simulink library "Renault_Library"  (Simulink 9.2)
settings: Solver=ode3  StartTime=0.0  StopTime=10.0  FixedStep=0.020  RelTol=1e-3  EnableMultiTasking=on
undefined symbols (not defined in the file or in its folder): ABC, AssystemProposal, b, chkval, CoeffA_C, CoeffB_C, CoeffMean_C, ConfTime_C, DiagDelay_C, DividePercent, FailureConfActive_C, FallingEdgeDuration_C, HighLevelThresholdVoltage_C, HighLevelVoltage_C, HighLevelVoltageTunable_C, InitValue_C, InputVoltRangeMax_C, InputVoltRangeMin_C, LowActiveContact_C, LowLevelThresholdVoltage_C, LowLevelVoltage_C, MaxOperatingVoltage_C, MinOperatingVoltage_C, mV2V_conversion, OUT1, OutOfRangeHigh_C, OutOfRangeLow_C, PinSupplySaturationMax, PinSupplySaturationMin, Res1Value_C, Res2Value_C, Res3Value_C, RisingEdgeDuration_C, RSA_CANSignalFailMonitoring, SampleTime_C, service14Table, service19Table, service22Table, service24Table, service28Table, service2ETable, service2FTable, service31Table, service87Table, SizeXStateDID2FTable, SizeXTable14, SizeXTable19, SizeXTable22, SizeXTable24, SizeXTable28, SizeXTable2E, SizeXTable2F, SizeXTable31, SizeXTable87, SizeYStateDID2FTable, SizeYTable14, SizeYTable19, SizeYTable22, SizeYTable24, SizeYTable28 ... (+22)
undefined symbols (not defined in the file): BatteryVoltage_ActivationThreshold_C, FrameStatus_AbsentValue_C, FrameStatus_ConfAbsentValue_C, MemThreshold_C, NAMEOFTHEMODEL_SAMPLE_TIME_CST, NbAbs1_C, NbConfAbs1_C, NbConfPres1_C, NbPres1_C, NOMDUSIGNAL_FaultConfTime_C, NOMDUSIGNAL_FaultRehabTime_C, NOMDUSIGNAL_switchvalue_C, NOMDUSIGNAL_unavailablevalue_C, PeriodFrame1_C, ResetTime_C, WakeUpTime_C
libraries: $bdroot x112, simulink x75
(part 10 of 32)

== system Renault_Library/Complex blocks/Math Operations/RSA_DivisionByZero_v01_04 ==
  den : Inport  Tag=AM,In,0,1,Name,C;CMP,Port,PortWidth,SampleTime;
  num : Inport  Tag=AM,In,0,1,Name,C;CMP,Port,PortWidth,SampleTime;  Port=2
  ans_of_0div : Inport  Tag=AM,In,0,1,Name,C;CMP,Port,PortWidth,SampleTime;  Port=3
  Divide : Product  Tag=[LIB]  Inputs=*/  OutDataTypeStr=Inherit: Inherit via back propagation  SaturateOnIntegerOverflow=on  RndMeth=Zero
      settings: AttributesFormatString=SAT = %<SaturateOnIntegerOverflow>
  RelationalOperator : RelationalOperator  Tag=AM,Std,2,1;CMP,Operator;  Operator=~=  OutDataTypeStr=boolean  RndMeth=Nearest  InputSameDT=on
  Switch : Switch  Tag=GEN,Threshold;AM,Switch,2,1;CMP,Threshold;  Criteria=u2 ~= 0  RndMeth=Zero  SaturateOnIntegerOverflow=on  InputSameDT=on
      settings: AttributesFormatString=Criteria=%<Criteria>
  imm_zero : Constant  Tag=AM,Constant,0,1,Value;CMP,Value;  Value=DIVBY0  OutDataTypeStr=Inherit: Inherit via back propagation  SampleTime=-1
  ans : Outport  Tag=AM,Out,1,0,Name,NC;CMP,Port,OutputWhenDisabled,InitialOutput;
  wires:
  den/1 -> RelationalOperator/1, Divide/2
  Switch/1 -> ans/1
  ans_of_0div/1 -> Switch/3
  imm_zero/1 -> RelationalOperator/2
  Divide/1 -> Switch/1
  RelationalOperator/1 -> Switch/2
  num/1 -> Divide/1

== system Renault_Library/Complex blocks/Math Operations/RSA_DivisionByZero_v01_05 ==
  den : Inport
      settings: AttributesFormatString=Type : %<OutDataTypeStr>
  num : Inport  Port=2
      settings: AttributesFormatString=Type : %<OutDataTypeStr>
  ans_of_0div : Inport  Port=3
      settings: AttributesFormatString=Type : %<OutDataTypeStr>
  Abs1 : Abs  OutDataTypeStr=Inherit: Inherit via back propagation  RndMeth=Zero  SaturateOnIntegerOverflow=on
  Divide : Product  Inputs=*/  OutDataTypeStr=Inherit: Inherit via back propagation  SaturateOnIntegerOverflow=on  RndMeth=Zero
      settings: AttributesFormatString=SAT = %<SaturateOnIntegerOverflow>
  RelationalOperator : RelationalOperator  Operator=>  OutDataTypeStr=boolean  RndMeth=Nearest  InputSameDT=on
  Switch : Switch  Criteria=u2 ~= 0  RndMeth=Zero  SaturateOnIntegerOverflow=on  InputSameDT=on
      settings: AttributesFormatString=Criteria=%<Criteria>
  imm_zero : Constant  Value=Den_Tol  OutDataTypeStr=Inherit: Inherit via back propagation
  result : Outport
      settings: AttributesFormatString=Type : %<OutDataTypeStr>\nInit :%<InitialOutput>
  wires:
  Abs1/1 -> RelationalOperator/1
  den/1 -> Abs1/1, Divide/2
  Switch/1 -> result/1
  ans_of_0div/1 -> Switch/3
  imm_zero/1 -> RelationalOperator/2
  Divide/1 -> Switch/1
  RelationalOperator/1 -> Switch/2
  num/1 -> Divide/1

== system Renault_Library/Complex blocks/Math Operations/RSA_Modulo_V01_00 ==
  note: Generate an error if inputs are not of the same data types
  X : Inport
  Y : Inport  Port=2
  Abs1 : Abs  RndMeth=Zero
  Abs2 : Abs  RndMeth=Zero
  Add1 : Sum  Inputs=-+  OutDataTypeStr=Inherit: Inherit via back propagation  RndMeth=Zero  InputSameDT=on
      settings: AttributesFormatString=SAT = %<SaturateOnIntegerOverflow>
  Add2 : Sum  Inputs=+-  OutDataTypeStr=Inherit: Inherit via back propagation  RndMeth=Zero  InputSameDT=on
      settings: AttributesFormatString=SAT = %<SaturateOnIntegerOverflow>
  Constant1 : Constant  Value=cmpTol  OutDataTypeStr=Inherit: Inherit via back propagation
  Constant2 : Constant  Value=cmpZero  OutDataTypeStr=Inherit: Inherit via back propagation
  Constant3 : Constant  Value=constOne  OutDataTypeStr=uint8
  Divide : Product  Inputs=*/  OutDataTypeStr=InternalDataType  SaturateOnIntegerOverflow=on  RndMeth=Zero
  LogicalOperator1 : Logic  OutDataTypeStr=boolean
  LogicalOperator3 : Logic  Operator=XOR  OutDataTypeStr=boolean
  Mux2 : Mux  Inputs=2
  Product1 : Product  OutDataTypeStr=Inherit: Inherit via back propagation  RndMeth=Zero
  Product2 : Product  OutDataTypeStr=Inherit: Inherit via back propagation  SaturateOnIntegerOverflow=on  RndMeth=Zero
  RelationalOperator1 : RelationalOperator  Operator=>  OutDataTypeStr=boolean  RndMeth=Nearest  InputSameDT=on
  RelationalOperator2 : RelationalOperator  Operator=>  OutDataTypeStr=boolean  RndMeth=Nearest  InputSameDT=on
  RelationalOperator5 : RelationalOperator  Operator=<  OutDataTypeStr=boolean  RndMeth=Nearest  InputSameDT=on
  RelationalOperator6 : RelationalOperator  Operator=<  OutDataTypeStr=boolean  RndMeth=Nearest  InputSameDT=on
  Sign : Signum
  Switch : Switch  Criteria=u2 ~= 0  RndMeth=Zero  InputSameDT=on
      settings: AttributesFormatString=Criteria=%<Criteria>
  Switch4 : Switch  Criteria=u2 ~= 0  RndMeth=Zero  InputSameDT=on
      settings: AttributesFormatString=Criteria=%<Criteria>
  Terminator1 : Terminator
  Terminator2 : Terminator
  Terminator3 : Terminator
  M : Outport
  wires:
  Add2/1 -> Switch4/1
  RelationalOperator1/1 -> Switch/2
  [absY] -> Add2/1
  [absX] -> Add1/2
  [absY] -> Product2/2
  Abs2/1 -> [absY]
  Abs1/1 -> [absX]
  Y/1 -> Abs2/1, [Y]
  X/1 -> [X], Abs1/1
  [cmpzero] -> RelationalOperator5/2
  [cmpzero] -> RelationalOperator6/2
  [Y] -> RelationalOperator5/1
  [X] -> RelationalOperator6/1
  RelationalOperator6/1 -> LogicalOperator3/1
  RelationalOperator5/1 -> LogicalOperator3/2
  Product2/1 -> Add1/1
  [X] -> Switch/3
  Switch/1 -> M/1
  [absX] -> Divide/1
  [absY] -> Divide/2
  [absY] -> RelationalOperator1/1
  [Y] -> Mux2/2
  [X] -> Mux2/1
  Mux2/1 -> Terminator3/1
  Constant1/1 -> [cmpTol]
  [cmpTol] -> RelationalOperator1/2
  Divide/1 -> Product2/1
  Add1/1 -> Add2/2, RelationalOperator2/1, Switch4/3
  Constant2/1 -> [cmpzero]
  [cmpzero] -> RelationalOperator2/2
  RelationalOperator2/1 -> LogicalOperator1/2
  LogicalOperator3/1 -> LogicalOperator1/1
  LogicalOperator1/1 -> Switch4/2
  [Y] -> Sign/1
  Sign/1 -> [Mulconst]
  Constant3/1 -> [constone]
  [constone] -> Terminator1/1
  [Mulconst] -> Terminator2/1
  [Mulconst] -> Product1/2
  Switch4/1 -> Product1/1
  Product1/1 -> Switch/1

== system Renault_Library/Complex blocks/Signal Routing/RSA_DataTypeDuplicate ==
  In1 : Inport
      settings: AttributesFormatString=Type : %<OutDataType>\nScaling : %<OutScaling>
  In2 : Inport  Port=2
      settings: AttributesFormatString=Type : %<OutDataType>\nScaling : %<OutScaling>
  In3 : Inport  Port=3
      settings: AttributesFormatString=Type : %<OutDataType>\nScaling : %<OutScaling>
  Mux : Mux  Inputs=3
  Terminator : Terminator
  wires:
  In3/1 -> Mux/3
  In2/1 -> Mux/2
  In1/1 -> Mux/1
  Mux/1 -> Terminator/1

== system Renault_Library/Complex blocks/Signal Routing/RSA_DataTypeDuplicate_v01_03 ==
  In1 : Inport
  In2 : Inport  Port=2
  In3 : Inport  Port=3
  Mux : Mux  Inputs=3
  Terminator : Terminator
  wires:
  Mux/1 -> Terminator/1
  In1/1 -> Mux/1
  In2/1 -> Mux/2
  In3/1 -> Mux/3

== system Renault_Library/Complex blocks/Signal Routing/RSA_SlopeSwitch2Inputs ==
  Switch_Cmd : Inport  Tag=AM,In,0,1,Name,C;CMP,Port,PortWidth,SampleTime;
      settings: AttributesFormatString=Type : %<OutDataTypeStr>
  Pos_Slope : Inport  Port=2
      settings: AttributesFormatString=Type : %<OutDataTypeStr>
  Neg_Slope : Inport  Port=3
      settings: AttributesFormatString=Type : %<OutDataTypeStr>
  In0 : Inport  Port=4
      settings: AttributesFormatString=Type : %<OutDataTypeStr>
  In1 : Inport  Port=5
      settings: AttributesFormatString=Type : %<OutDataTypeStr>
  Constant : Constant  Value=STHCMD  OutDataTypeStr=Inherit: Inherit via back propagation  SampleTime=-1
  Constant1 : Constant  Value=st  OutDataTypeStr=Inherit: Inherit via back propagation  SampleTime=-1
  DataTypeConversion : DataTypeConversion  RndMeth=Zero
  DataTypeConversion1 : DataTypeConversion  RndMeth=Zero
  Multiport_Switch : MultiPortSwitch  Inputs=2  RndMeth=Zero  SaturateOnIntegerOverflow=on  InputSameDT=on
      settings: AttributesFormatString=PortOrder=%<DataPortOrder>
  Product : Product  OutDataTypeStr=Inherit: Inherit via back propagation  SaturateOnIntegerOverflow=on  RndMeth=Zero
      settings: AttributesFormatString=SAT = %<SaturateOnIntegerOverflow>
  Product1 : Product  OutDataTypeStr=Inherit: Inherit via back propagation  SaturateOnIntegerOverflow=on  RndMeth=Zero
      settings: AttributesFormatString=SAT = %<SaturateOnIntegerOverflow>
  Signal_Change_Compute : Subsystem
  Slope_On_Block : Subsystem
  Slope_On_Compute : Subsystem
  Sum : Sum  OutDataTypeStr=Inherit: Inherit via back propagation  RndMeth=Zero  InputSameDT=on
      settings: AttributesFormatString=SAT = %<SaturateOnIntegerOverflow>
  Switch2 : Switch  Tag=GEN,Threshold;AM,Switch,2,1;CMP,Threshold;  Criteria=u2 ~= 0  RndMeth=Zero  SaturateOnIntegerOverflow=on  InputSameDT=on
      settings: AttributesFormatString=Criteria=%<Criteria>
  UnitDelay1 : UnitDelay  InitialCondition=false  SampleTime=-1
  UnitDelay2 : UnitDelay  InitialCondition=SS2ININI  SampleTime=-1
  Output : Outport  Tag=AM,Out,1,0,Name,NC;CMP,Port,OutputWhenDisabled,InitialOutput;
      settings: AttributesFormatString=Type : %<OutDataTypeStr>\nInit :%<InitialOutput>
  Slope_On : Outport  Tag=AM,Out,1,0,Name,NC;CMP,Port,OutputWhenDisabled,InitialOutput;  Port=2
      settings: AttributesFormatString=Type : %<OutDataTypeStr>\nInit :%<InitialOutput>
  wires:
  DataTypeConversion1/1 -> Output/1
  DataTypeConversion/1 -> Slope_On/1
  Switch_Cmd/1 -> Sum/1
  Sum/1 -> Signal_Change_Compute/1(Switch_Cmd), Multiport_Switch/1
  Constant/1 -> Sum/2
  Pos_Slope/1 -> [Pos_Slope]
  Neg_Slope/1 -> [Neg_Slope]
  [Enable_Slope_On_Block] -> Switch2/2
  [Output_Not_Equals_Input] -> UnitDelay1/1
  Slope_On_Block/1(Output_Not_Equals_Input) -> [Output_Not_Equals_Input]
  Constant1/1 -> Product1/2, Product/2
  Multiport_Switch/1 -> [Input]
  [Input] -> Switch2/3
  [Input] -> Slope_On_Block/2(Input)
  Signal_Change_Compute/1(Signal_Change) -> Slope_On_Compute/1(Signal_Change), Slope_On_Block/1(Signal_Change)
  [Neg_Slope] -> Product1/1
  [Pos_Slope] -> Product/1
  Product1/1 -> Slope_On_Block/4(Neg_Slope)
  Product/1 -> Slope_On_Block/3(Pos_Slope)
  UnitDelay2/1 -> Slope_On_Block/5(Prev_Output)  "X2"
  UnitDelay1/1 -> Slope_On_Compute/2(Output_Not_Equals_Input)
  Switch2/1 -> DataTypeConversion1/1, UnitDelay2/1
  Slope_On_Block/2(Output) -> Switch2/1  "X1"
  Slope_On_Compute/1(Slope_On) -> [Enable_Slope_On_Block], Slope_On_Block/Enable, DataTypeConversion/1
  In0/1 -> Multiport_Switch/2
  In1/1 -> Multiport_Switch/3

== system Renault_Library/Complex blocks/Timers & Counters/RSA_IncAndDecCounter ==
  INC_COUNTER : Inport
      settings: AttributesFormatString=Type : %<OutDataTypeStr>
  DEC_COUNTER : Inport  Port=2
      settings: AttributesFormatString=Type : %<OutDataTypeStr>
  MAX_COUNTER : Inport  Port=3
      settings: AttributesFormatString=Type : %<OutDataTypeStr>
  MIN_COUNTER : Inport  Port=4
      settings: AttributesFormatString=Type : %<OutDataTypeStr>
  RESET_COUNTER : Inport  Port=5
      settings: AttributesFormatString=Type : %<OutDataTypeStr>
  COUNTER_STEP : Inport  Port=6
      settings: AttributesFormatString=Type : %<OutDataTypeStr>
  INIT_COUNTER : Inport  Port=7
      settings: AttributesFormatString=Type : %<OutDataTypeStr>
  Add1 : Sum  OutDataTypeStr=Inherit: Inherit via back propagation  RndMeth=Zero  SaturateOnIntegerOverflow=on  InputSameDT=on
      settings: AttributesFormatString=SAT = %<SaturateOnIntegerOverflow>
  Add2 : Sum  Inputs=+-  OutDataTypeStr=Inherit: Inherit via back propagation  RndMeth=Zero  SaturateOnIntegerOverflow=on  InputSameDT=on
      settings: AttributesFormatString=SAT = %<SaturateOnIntegerOverflow>
  Constant1 : Constant  Value=4  OutDataTypeStr=Inherit: Inherit via back propagation  SampleTime=-1
  Constant2 : Constant  Value=2  OutDataTypeStr=Inherit: Inherit via back propagation  SampleTime=-1
  Constant3 : Constant  Value=0  OutDataTypeStr=Inherit: Inherit via back propagation  SampleTime=-1
  Constant5 : Constant  Value=3  OutDataTypeStr=Inherit: Inherit via back propagation  SampleTime=-1
  Constant6 : Constant  OutDataTypeStr=uint8  SampleTime=-1
  Data_Type_Conversion : DataTypeConversion  RndMeth=Zero
  MinMax : MinMax  Inputs=2  OutDataTypeStr=Inherit: Inherit via back propagation  RndMeth=Zero  InputSameDT=on
  MinMax1 : MinMax  Function=max  Inputs=2  OutDataTypeStr=Inherit: Inherit via back propagation  RndMeth=Zero  InputSameDT=on
  Multiport_Switch : MultiPortSwitch  Inputs=4  RndMeth=Zero  InputSameDT=on
      settings: AttributesFormatString=PortOrder=%<DataPortOrder>
  Switch{,2..4} (4 alike) : Switch  Criteria=u2 ~= 0  RndMeth=Zero  InputSameDT=on
      settings: AttributesFormatString=Criteria=%<Criteria>
  UnitDelay : UnitDelay  InitialCondition=true  SampleTime=-1
      settings: AttributesFormatString=IC = %<X0>
  Unit_Delay1 : UnitDelay  SampleTime=-1
      settings: AttributesFormatString=IC = %<X0>
  true : Constant  Value=false  OutDataTypeStr=boolean  SampleTime=-1
  COUNTER_OUT : Outport
      settings: AttributesFormatString=Type : %<OutDataTypeStr>\nInit :%<InitialOutput>
  wires:
  [MIN_COUNTER] -> MinMax1/2
  MinMax/1 -> MinMax1/1
  Multiport_Switch/1 -> MinMax/1
  [MAX_COUNTER] -> MinMax/2
  Constant2/1 -> Switch2/1
  Constant6/1 -> Switch4/1
  Switch2/1 -> Switch4/3
  Switch4/1 -> [SELECT_COUNTER]
  [SELECT_COUNTER] -> Multiport_Switch/1
  Add1/1 -> Multiport_Switch/2
  Add2/1 -> Multiport_Switch/3
  [COUNTER_STEP] -> Add2/2
  [COUNTER_STEP] -> Add1/2
  Unit_Delay1/1 -> Add1/1, Add2/1, Multiport_Switch/5
  MAX_COUNTER/1 -> [MAX_COUNTER]
  MIN_COUNTER/1 -> [MIN_COUNTER]
  INC_COUNTER/1 -> Switch4/2
  DEC_COUNTER/1 -> Switch2/2
  Constant5/1 -> Switch3/1
  Constant1/1 -> Switch3/3
  Switch3/1 -> Switch2/3
  RESET_COUNTER/1 -> Switch3/2
  Constant3/1 -> Multiport_Switch/4
  COUNTER_STEP/1 -> [COUNTER_STEP]
  Data_Type_Conversion/1 -> COUNTER_OUT/1
  true/1 -> UnitDelay/1
  INIT_COUNTER/1 -> [INIT_COUNTER]
  [INIT_COUNTER] -> Switch/1
  MinMax1/1 -> Switch/3
  Switch/1 -> Unit_Delay1/1, Data_Type_Conversion/1
  UnitDelay/1 -> Switch/2

== system Renault_Library/Complex blocks/Timers & Counters/RSA_IncAndDecCounter_V01_01 ==
  INC_COUNTER : Inport
      settings: AttributesFormatString=Type : %<OutDataTypeStr>
  DEC_COUNTER : Inport  Port=2
      settings: AttributesFormatString=Type : %<OutDataTypeStr>
  MAX_COUNTER : Inport  Port=3
      settings: AttributesFormatString=Type : %<OutDataTypeStr>
  MIN_COUNTER : Inport  Port=4
      settings: AttributesFormatString=Type : %<OutDataTypeStr>
  RESET_COUNTER : Inport  Port=5
      settings: AttributesFormatString=Type : %<OutDataTypeStr>
  COUNTER_STEP : Inport  Port=6
      settings: AttributesFormatString=Type : %<OutDataTypeStr>
  INIT_COUNTER : Inport  Port=7
      settings: AttributesFormatString=Type : %<OutDataTypeStr>
  Add1 : Sum  OutDataTypeStr=Inherit: Inherit via back propagation  RndMeth=Zero  SaturateOnIntegerOverflow=on  InputSameDT=on
      settings: AttributesFormatString=SAT = %<SaturateOnIntegerOverflow>
  Add2 : Sum  Inputs=+-  OutDataTypeStr=Inherit: Inherit via back propagation  RndMeth=Zero  SaturateOnIntegerOverflow=on  InputSameDT=on
      settings: AttributesFormatString=SAT = %<SaturateOnIntegerOverflow>
  Constant2 : Constant  Value=CONS_TWO  OutDataTypeStr=Inherit: Inherit via back propagation
  Constant3 : Constant  Value=CONS_ZERO  OutDataTypeStr=Inherit: Inherit via back propagation
  Constant4 : Constant  Value=CONS_ZERO  OutDataTypeStr=uint8
  Constant5 : Constant  Value=CONS_THREE  OutDataTypeStr=Inherit: Inherit via back propagation
  Constant6 : Constant  Value=CONS_ONE  OutDataTypeStr=uint8
  Data_Type_Conversion : DataTypeConversion  RndMeth=Zero
  MinMax : MinMax  Inputs=2  OutDataTypeStr=Inherit: Inherit via back propagation  RndMeth=Zero  InputSameDT=on
  MinMax1 : MinMax  Function=max  Inputs=2  OutDataTypeStr=Inherit: Inherit via back propagation  RndMeth=Zero  InputSameDT=on
  Multiport_Switch : MultiPortSwitch  DataPortOrder=Zero-based contiguous  Inputs=4  zeroidx=on  RndMeth=Zero  InputSameDT=on
      settings: AttributesFormatString=PortOrder=%<DataPortOrder>
  Switch{,2..4} (4 alike) : Switch  Criteria=u2 ~= 0  RndMeth=Zero  InputSameDT=on
      settings: AttributesFormatString=Criteria=%<Criteria>
  UnitDelay : UnitDelay  InitialCondition=true  SampleTime=-1
      settings: AttributesFormatString=IC = %<X0>
  Unit_Delay1 : UnitDelay  SampleTime=-1
      settings: AttributesFormatString=IC = %<X0>
  true : Constant  Value=false  OutDataTypeStr=boolean
  COUNTER_OUT : Outport
      settings: AttributesFormatString=Type : %<OutDataTypeStr>\nInit :%<InitialOutput>
  wires:
  Constant5/1 -> Switch3/3
  Constant2/1 -> Switch3/1
  Constant6/1 -> Switch2/1
  Constant4/1 -> Switch4/1
  [MIN_COUNTER] -> MinMax1/2
  MinMax/1 -> MinMax1/1
  Multiport_Switch/1 -> MinMax/1
  [MAX_COUNTER] -> MinMax/2
  Switch2/1 -> Switch4/3
  Switch4/1 -> [SELECT_COUNTER]
  [SELECT_COUNTER] -> Multiport_Switch/1
  Add1/1 -> Multiport_Switch/2
  Add2/1 -> Multiport_Switch/3
  [COUNTER_STEP] -> Add2/2
  [COUNTER_STEP] -> Add1/2
  Unit_Delay1/1 -> Add1/1, Add2/1, Multiport_Switch/5
  MAX_COUNTER/1 -> [MAX_COUNTER]
  MIN_COUNTER/1 -> [MIN_COUNTER]
  INC_COUNTER/1 -> Switch4/2
  DEC_COUNTER/1 -> Switch2/2
  Switch3/1 -> Switch2/3
  RESET_COUNTER/1 -> Switch3/2
  Constant3/1 -> Multiport_Switch/4
  COUNTER_STEP/1 -> [COUNTER_STEP]
  Data_Type_Conversion/1 -> COUNTER_OUT/1
  true/1 -> UnitDelay/1
  INIT_COUNTER/1 -> [INIT_COUNTER]
  [INIT_COUNTER] -> Switch/1
  MinMax1/1 -> Switch/3
  Switch/1 -> Unit_Delay1/1, Data_Type_Conversion/1
  UnitDelay/1 -> Switch/2

== system Renault_Library/Complex blocks/Timers & Counters/RSA_Timer ==
  Init : Inport
      settings: AttributesFormatString=Type : %<OutDataTypeStr>
  Duration : Inport  Port=2
      settings: AttributesFormatString=Type : %<OutDataTypeStr>
  Constant1 : Constant  Tag=AM,Constant,0,1,Value;CMP,Value;  Value=TIM1INI  OutDataTypeStr=Inherit: Inherit via back propagation  SampleTime=-1
  Constant2 : Constant  Tag=AM,Constant,0,1,Value;CMP,Value;  Value=TIM1INI  OutDataTypeStr=Inherit: Inherit via back propagation  SampleTime=-1
  Constant3 : Constant  Tag=AM,Constant,0,1,Value;CMP,Value;  Value=st  OutDataTypeStr=Inherit: Inherit via back propagation  SampleTime=-1
  Constant4 : Constant  Tag=AM,Constant,0,1,Value;CMP,Value;  Value=TIM1INI  OutDataTypeStr=Inherit: Inherit via back propagation  SampleTime=-1
  DataTypeConversion : DataTypeConversion  RndMeth=Zero
  DelayInput1 : UnitDelay  Description=\nStore in Global RAM  InitialCondition=timeoutinit  SampleTime=-1
  DelayInput2 : UnitDelay  Description=\nStore in Global RAM  InitialCondition=TIM1INI  SampleTime=-1
  EdgeRising : Subsystem  mask(EdgeRising){vinit=initinit}
      mask prompts: vinit "Initialisation"
      mask help: When a rising edge is used as input, the ouput is equal to one during the fall of the signal. Otherwise the output is zero.
      mask Display:
      | plot([0.2 0.4 0.4 0.6],[0.6 0.6 0.9 0.9]);
      | plot([0.2 0.4 0.4 0.4 0.6],[0.1 0.1 0.4 0.1 0.1])
  LogicalOperator : Logic  OutDataTypeStr=boolean
  RelationalOperator1 : RelationalOperator  OutDataTypeStr=boolean  ZeroCross=off  RndMeth=Nearest  InputSameDT=on
  RelationalOperator2 : RelationalOperator  Operator=<=  OutDataTypeStr=boolean  ZeroCross=off  RndMeth=Nearest  InputSameDT=on
  Sum : Sum  Inputs=+-  OutDataTypeStr=Inherit: Inherit via back propagation  RndMeth=Zero  SaturateOnIntegerOverflow=on  InputSameDT=on
      settings: AttributesFormatString=SAT = %<SaturateOnIntegerOverflow>
  Switch{1..4} (4 alike) : Switch  Tag=GEN,Threshold;AM,Switch,2,1;CMP,Threshold;  Criteria=u2 ~= 0  RndMeth=Zero  SaturateOnIntegerOverflow=on  InputSameDT=on
      settings: AttributesFormatString=Criteria=%<Criteria>
  Timeout : Outport
      settings: AttributesFormatString=Type : %<OutDataTypeStr>\nInit :%<InitialOutput>
  Timer : Outport  Port=2
      settings: AttributesFormatString=Type : %<OutDataTypeStr>\nInit :%<InitialOutput>
  wires:
  Constant2/1 -> Switch4/3
  DelayInput2/1 -> RelationalOperator2/2, Sum/1  "X2"
  RelationalOperator2/1 -> Switch4/2
  Sum/1 -> Switch4/1
  Switch4/1 -> Switch2/3
  Duration/1 -> Switch2/1
  [EdgeRising] -> Switch2/2
  Constant3/1 -> Sum/2, RelationalOperator2/1
  Switch3/1 -> DelayInput2/1, DataTypeConversion/1  "X5"
  Constant1/1 -> Switch3/1
  RelationalOperator1/1 -> LogicalOperator/2, Switch3/2  "X4"
  DelayInput1/1 -> Switch1/3
  Switch1/1 -> LogicalOperator/1, DelayInput1/1  "Flag_of_first_initialisation"
  LogicalOperator/1 -> Timeout/1
  Constant4/1 -> RelationalOperator1/1
  Switch2/1 -> Switch3/3, RelationalOperator1/2  "X3"
  Init/1 -> EdgeRising/1(In)
  EdgeRising/1(Out) -> [EdgeRising], Switch1/1, Switch1/2
  DataTypeConversion/1 -> Timer/1

== system Renault_Library/Complex blocks/Timers & Counters/RSA_Timer1 ==
  Init : Inport
      settings: AttributesFormatString=Type : %<OutDataTypeStr>
  Duration : Inport  Port=2
      settings: AttributesFormatString=Type : %<OutDataTypeStr>
  Timestep : Inport  Port=3
      settings: AttributesFormatString=Type : %<OutDataTypeStr>
  Constant1 : Constant  Tag=AM,Constant,0,1,Value;CMP,Value;  Value=TIM1INI  OutDataTypeStr=Inherit: Inherit via back propagation  SampleTime=-1
  Constant2 : Constant  Tag=AM,Constant,0,1,Value;CMP,Value;  Value=TIM1INI  OutDataTypeStr=Inherit: Inherit via back propagation  SampleTime=-1
  Constant4 : Constant  Tag=AM,Constant,0,1,Value;CMP,Value;  Value=TIM1INI  OutDataTypeStr=Inherit: Inherit via back propagation  SampleTime=-1
  DataTypeConversion : DataTypeConversion  RndMeth=Zero
  DelayInput1 : UnitDelay  Description=\nStore in Global RAM  InitialCondition=timeoutinit  SampleTime=-1
  DelayInput2 : UnitDelay  Description=\nStore in Global RAM  InitialCondition=TIM1INI  SampleTime=-1
  EdgeRising : Subsystem  mask(EdgeRising){vinit=initinit}
      mask prompts: vinit "Initialisation"
      mask help: When a rising edge is used as input, the ouput is equal to one during the fall of the signal. Otherwise the output is zero.
      mask Display:
      | plot([0.2 0.4 0.4 0.6],[0.6 0.6 0.9 0.9]);
      | plot([0.2 0.4 0.4 0.4 0.6],[0.1 0.1 0.4 0.1 0.1])
  LogicalOperator : Logic  OutDataTypeStr=boolean
  RelationalOperator1 : RelationalOperator  OutDataTypeStr=boolean  ZeroCross=off  RndMeth=Nearest  InputSameDT=on
  RelationalOperator2 : RelationalOperator  Operator=<=  OutDataTypeStr=boolean  ZeroCross=off  RndMeth=Nearest  InputSameDT=on
  Sum : Sum  Inputs=+-  OutDataTypeStr=Inherit: Inherit via back propagation  RndMeth=Zero  SaturateOnIntegerOverflow=on  InputSameDT=on
      settings: AttributesFormatString=SAT = %<SaturateOnIntegerOverflow>
  Switch{1..4} (4 alike) : Switch  Tag=GEN,Threshold;AM,Switch,2,1;CMP,Threshold;  Criteria=u2 ~= 0  RndMeth=Zero  SaturateOnIntegerOverflow=on  InputSameDT=on
      settings: AttributesFormatString=Criteria=%<Criteria>
  Timeout : Outport
      settings: AttributesFormatString=Type : %<OutDataTypeStr>\nInit :%<InitialOutput>
  Timer : Outport  Port=2
      settings: AttributesFormatString=Type : %<OutDataTypeStr>\nInit :%<InitialOutput>
  wires:
  [Timestep] -> RelationalOperator2/1, Sum/2
  [EdgeRising] -> Switch2/2
  EdgeRising/1(Out) -> Switch1/2, Switch1/1, [EdgeRising]
  Init/1 -> EdgeRising/1(In)
  Constant4/1 -> RelationalOperator1/1
  LogicalOperator/1 -> Timeout/1
  Switch1/1 -> DelayInput1/1, LogicalOperator/1
  DelayInput1/1 -> Switch1/3
  Timestep/1 -> [Timestep]
  RelationalOperator1/1 -> Switch3/2, LogicalOperator/2
  Constant1/1 -> Switch3/1
  Switch3/1 -> DelayInput2/1, DataTypeConversion/1
  DataTypeConversion/1 -> Timer/1
  Switch2/1 -> RelationalOperator1/2, Switch3/3
  Duration/1 -> Switch2/1
  Switch4/1 -> Switch2/3
  Sum/1 -> Switch4/1
  RelationalOperator2/1 -> Switch4/2
  DelayInput2/1 -> Sum/1, RelationalOperator2/2
  Constant2/1 -> Switch4/3

== system Renault_Library/Complex blocks/Timers & Counters/RSA_Timer1_v01_04 ==
  Init : Inport
      settings: AttributesFormatString=Type : %<OutDataTypeStr>
  Duration : Inport  Port=2
      settings: AttributesFormatString=Type : %<OutDataTypeStr>
  Timestep : Inport  Port=3
      settings: AttributesFormatString=Type : %<OutDataTypeStr>
  Constant1 : Constant  Tag=AM,Constant,0,1,Value;CMP,Value;  Value=TIM1INI  OutDataTypeStr=Inherit: Inherit via back propagation  SampleTime=-1
  Constant3 : Constant  Tag=AM,Constant,0,1,Value;CMP,Value;  Value=TIM1INI  OutDataTypeStr=Inherit: Inherit via back propagation  SampleTime=-1
  DataTypeConversion : DataTypeConversion  RndMeth=Zero
  DelayInput1 : UnitDelay  Description=\nStore in Global RAM  InitialCondition=timeoutinit  SampleTime=-1
  DelayInput2 : UnitDelay  Description=\nStore in Global RAM  InitialCondition=TIM1INI  SampleTime=-1
  EdgeRising : Subsystem  mask(EdgeRising){vinit=initinit}
      mask prompts: vinit "Initialisation"
      mask help: When a rising edge is used as input, the ouput is equal to one during the fall of the signal. Otherwise the output is zero.
      mask Display:
      | plot([0.2 0.4 0.4 0.6],[0.6 0.6 0.9 0.9]);
      | plot([0.2 0.4 0.4 0.4 0.6],[0.1 0.1 0.4 0.1 0.1])
  LogicalOperator : Logic  OutDataTypeStr=boolean
  RelationalOperator1 : RelationalOperator  Operator=>  OutDataTypeStr=boolean  ZeroCross=off  RndMeth=Nearest  InputSameDT=on
  RelationalOperator2 : RelationalOperator  Operator=<=  OutDataTypeStr=boolean  ZeroCross=off  RndMeth=Nearest  InputSameDT=on
  Sum : Sum  Inputs=+-  OutDataTypeStr=Inherit: Inherit via back propagation  RndMeth=Zero  SaturateOnIntegerOverflow=on  InputSameDT=on
      settings: AttributesFormatString=SAT = %<SaturateOnIntegerOverflow>
  Switch{1..4} (4 alike) : Switch  Tag=GEN,Threshold;AM,Switch,2,1;CMP,Threshold;  Criteria=u2 ~= 0  RndMeth=Zero  SaturateOnIntegerOverflow=on  InputSameDT=on
      settings: AttributesFormatString=Criteria=%<Criteria>
  Timeout : Outport
      settings: AttributesFormatString=Type : %<OutDataTypeStr>\nInit :%<InitialOutput>
  Timer : Outport  Port=2
      settings: AttributesFormatString=Type : %<OutDataTypeStr>\nInit :%<InitialOutput>
  wires:
  [Timestep] -> RelationalOperator2/1, Sum/2
  [EdgeRising] -> Switch2/2
  EdgeRising/1(Out) -> Switch1/2, Switch1/1, [EdgeRising]
  Init/1 -> EdgeRising/1(In)
  LogicalOperator/1 -> Timeout/1
  Switch1/1 -> DelayInput1/1, LogicalOperator/1
  DelayInput1/1 -> Switch1/3
  Timestep/1 -> [Timestep]
  RelationalOperator1/1 -> LogicalOperator/2, Switch3/2
  Switch3/1 -> DataTypeConversion/1, DelayInput2/1
  DataTypeConversion/1 -> Timer/1
  Switch2/1 -> Switch3/3, RelationalOperator1/2
  Switch4/1 -> Switch2/3
  Sum/1 -> Switch4/1
  RelationalOperator2/1 -> Switch4/2
  DelayInput2/1 -> Sum/1, RelationalOperator2/2
  Duration/1 -> Switch2/1
  [Timestep] -> RelationalOperator1/1
  Constant3/1 -> Switch3/1
  Constant1/1 -> Switch4/3

== system Renault_Library/Complex blocks/Timers & Counters/RSA_Timer1_v01_05 ==
  Init : Inport
      settings: AttributesFormatString=Type : %<OutDataTypeStr>
  Duration : Inport  Port=2
      settings: AttributesFormatString=Type : %<OutDataTypeStr>
  Timestep : Inport  Port=3
      settings: AttributesFormatString=Type : %<OutDataTypeStr>
  Constant1 : Constant  Tag=AM,Constant,0,1,Value;CMP,Value;  Value=TIM1INI  OutDataTypeStr=Inherit: Inherit via back propagation  SampleTime=-1
  Constant3 : Constant  Tag=AM,Constant,0,1,Value;CMP,Value;  Value=TIM1INI  OutDataTypeStr=Inherit: Inherit via back propagation  SampleTime=-1
  Constant4 : Constant  Tag=AM,Constant,0,1,Value;CMP,Value;  Value=1000  OutDataTypeStr=uint16  SampleTime=-1
  Constant5 : Constant  Tag=AM,Constant,0,1,Value;CMP,Value;  OutDataTypeStr=Inherit: Inherit via back propagation  SampleTime=-1
  DataTypeConversion : DataTypeConversion  RndMeth=Zero
  DataTypeConversion1 : DataTypeConversion  OutDataTypeStr=uint32  SaturateOnIntegerOverflow=on  RndMeth=Zero
  DataTypeConversion2 : DataTypeConversion  RndMeth=Zero
  DelayInput1 : UnitDelay  Description=\nStore in Global RAM  InitialCondition=timeoutinit  SampleTime=-1
      settings: AttributesFormatString=IC = %<X0>
  DelayInput2 : UnitDelay  Description=\nStore in Global RAM  InitialCondition=TIM1INI  SampleTime=-1
      settings: AttributesFormatString=IC = %<X0>
  EdgeRising : Subsystem  mask(EdgeRising){vinit=initinit}
      mask prompts: vinit "Initialisation"
      mask help: When a rising edge is used as input, the ouput is equal to one during the fall of the signal. Otherwise the output is zero.
      mask Display:
      | plot([0.2 0.4 0.4 0.6],[0.6 0.6 0.9 0.9]);
      | plot([0.2 0.4 0.4 0.4 0.6],[0.1 0.1 0.4 0.1 0.1])
  LogicalOperator : Logic  OutDataTypeStr=boolean
  Product : Product  SaturateOnIntegerOverflow=on  RndMeth=Zero
  Product1 : Product  SaturateOnIntegerOverflow=on  RndMeth=Zero
  Product2 : Product  Inputs=*/  OutDataTypeStr=Inherit: Inherit via back propagation  SaturateOnIntegerOverflow=on  RndMeth=Zero
  RSA_CompareToZero : $bdroot/Complex blocks/Logic & Bit Operations/RSA_CompareToZero  Tag=[LIB]  SystemSampleTime=-1  relop=~=  LogicOutDataTypeMode=boolean
      settings: AttributesFormatString=%<MaskType> : Ver : 01.03\nOperator: %<relop>\nOutput data type mode: %<LogicOutDataTypeMode>\nEnable zero-crossing detection %<ZeroCross>
  RSA_DataTypeDuplicate_v01_03 : $bdroot/Complex blocks/Signal Routing/RSA_DataTypeDuplicate_v01_03  Tag=[LIB]  SystemSampleTime=-1  NoIn=2
      settings: AttributesFormatString=%<MaskType> : Ver : 01.03\nNo Of Inputs %<NoIn>
  RelationalOperator1 : RelationalOperator  Operator=>  OutDataTypeStr=boolean  ZeroCross=off  RndMeth=Nearest  InputSameDT=on
  RelationalOperator2 : RelationalOperator  Operator=<=  OutDataTypeStr=boolean  ZeroCross=off  RndMeth=Nearest  InputSameDT=on
  Subtract4 : Sum  Inputs=-+  OutDataTypeStr=Inherit: Inherit via back propagation  RndMeth=Zero  SaturateOnIntegerOverflow=on  InputSameDT=on
      settings: AttributesFormatString=SAT = %<SaturateOnIntegerOverflow>
  Sum : Sum  Inputs=+-  OutDataTypeStr=Inherit: Inherit via back propagation  RndMeth=Zero  SaturateOnIntegerOverflow=on  InputSameDT=on
      settings: AttributesFormatString=SAT = %<SaturateOnIntegerOverflow>
  Switch{1..5} (5 alike) : Switch  Tag=GEN,Threshold;AM,Switch,2,1;CMP,Threshold;  Criteria=u2 ~= 0  RndMeth=Zero  SaturateOnIntegerOverflow=on  InputSameDT=on
      settings: AttributesFormatString=Criteria=%<Criteria>
  Timeout : Outport
      settings: AttributesFormatString=Type : %<OutDataTypeStr>\nInit :%<InitialOutput>
  Timer : Outport  Port=2
      settings: AttributesFormatString=Type : %<OutDataTypeStr>\nInit :%<InitialOutput>
  wires:
  [Timestep1] -> RelationalOperator2/1, Sum/2
  [EdgeRising] -> Switch2/2
  EdgeRising/1(Out) -> Switch1/2, Switch1/1, [EdgeRising]
  Init/1 -> EdgeRising/1(In)
  LogicalOperator/1 -> Timeout/1
  Switch1/1 -> DelayInput1/1, LogicalOperator/1
  DelayInput1/1 -> Switch1/3
  RelationalOperator1/1 -> LogicalOperator/2, Switch3/2
  Switch3/1 -> DelayInput2/1, Product2/1
  DataTypeConversion/1 -> Timer/1
  Switch2/1 -> Switch3/3, RelationalOperator1/2
  Switch4/1 -> Switch2/3
  Sum/1 -> Switch4/1
  RelationalOperator2/1 -> Switch4/2
  DelayInput2/1 -> Sum/1, RelationalOperator2/2
  [Timestep1] -> RelationalOperator1/1
  Constant3/1 -> Switch3/1
  Constant1/1 -> Switch4/3
  [Timestep] -> DataTypeConversion1/1, Subtract4/2
  DataTypeConversion1/1 -> DataTypeConversion2/1
  DataTypeConversion2/1 -> Subtract4/1
  RSA_CompareToZero/1 -> Switch5/2
  Subtract4/1 -> RSA_CompareToZero/1
  Constant5/1 -> Switch5/3
  Constant4/1 -> Switch5/1
  Switch5/1 -> [tolcons]
  [tolcons] -> Product/2
  Product/1 -> [Timestep1]
  Duration/1 -> RSA_DataTypeDuplicate_v01_03/2, Product1/1
  [tolcons] -> Product1/2
  Product1/1 -> Switch2/1
  Timestep/1 -> RSA_DataTypeDuplicate_v01_03/1, [Timestep], Product/1
  [tolcons] -> Product2/2
  Product2/1 -> DataTypeConversion/1

== system Renault_Library/Complex blocks/Timers & Counters/RSA_Timer1_v01_06 ==
  Init : Inport
      settings: AttributesFormatString=Type : %<OutDataTypeStr>
  Duration : Inport  Port=2
      settings: AttributesFormatString=Type : %<OutDataTypeStr>
  Timestep : Inport  Port=3
      settings: AttributesFormatString=Type : %<OutDataTypeStr>
  Constant1 : Constant  Tag=AM,Constant,0,1,Value;CMP,Value;  Value=TIM1INI  OutDataTypeStr=Inherit: Inherit via back propagation  SampleTime=-1
  Constant2 : Constant  Tag=AM,Constant,0,1,Value;CMP,Value;  Value=CONS_ONE  OutDataTypeStr=Inherit: Inherit via back propagation
  Constant3 : Constant  Tag=AM,Constant,0,1,Value;CMP,Value;  Value=TIM1INI  OutDataTypeStr=Inherit: Inherit via back propagation  SampleTime=-1
  Constant4 : Constant  Tag=AM,Constant,0,1,Value;CMP,Value;  Value=Boosting_Decimal  OutDataTypeStr=uint32
  Constant5 : Constant  Tag=AM,Constant,0,1,Value;CMP,Value;  Value=Boosting_Whole  OutDataTypeStr=Inherit: Inherit via back propagation
  Constant6 : Constant  Tag=AM,Constant,0,1,Value;CMP,Value;  Value=CONS_ZERO  OutDataTypeStr=Inherit: Inherit via back propagation
  DataTypeConversion2 : DataTypeConversion  RndMeth=Zero
  DelayInput1 : UnitDelay  Description=\nStore in Global RAM  InitialCondition=timeoutinit  SampleTime=-1
      settings: AttributesFormatString=IC = %<X0>
  DelayInput2 : UnitDelay  Description=\nStore in Global RAM  InitialCondition=TIM1INI  SampleTime=-1
      settings: AttributesFormatString=IC = %<X0>
  EdgeRising : Subsystem  mask(EdgeRising){vinit=initinit}
      mask prompts: vinit "Initialisation"
      mask help: When a rising edge is used as input, the ouput is equal to one during the fall of the signal. Otherwise the output is zero.
      mask Display:
      | plot([0.2 0.4 0.4 0.6],[0.6 0.6 0.9 0.9]);
      | plot([0.2 0.4 0.4 0.4 0.6],[0.1 0.1 0.4 0.1 0.1])
  LogicalOperator : Logic  OutDataTypeStr=boolean
  Product : Product  OutDataTypeStr=Inherit: Inherit via back propagation  SaturateOnIntegerOverflow=on  RndMeth=Zero
  Product1 : Product  OutDataTypeStr=Inherit: Inherit via back propagation  SaturateOnIntegerOverflow=on  RndMeth=Zero
  Product2 : Product  Inputs=*/  OutDataTypeStr=Inherit: Inherit via back propagation  SaturateOnIntegerOverflow=on  RndMeth=Zero
  Product3 : Product  OutDataTypeStr=uint32  SaturateOnIntegerOverflow=on  RndMeth=Zero
  RSA_DataTypeDuplicate_v01_03 : $bdroot/Complex blocks/Signal Routing/RSA_DataTypeDuplicate_v01_03  Tag=[LIB]  SystemSampleTime=-1  NoIn=2
      settings: AttributesFormatString=%<MaskType> : Ver : 01.03\nNo Of Inputs %<NoIn>
  RelationalOperator1 : RelationalOperator  Operator=>  OutDataTypeStr=boolean  ZeroCross=off  RndMeth=Nearest  InputSameDT=on
  RelationalOperator2 : RelationalOperator  Operator=<=  OutDataTypeStr=boolean  ZeroCross=off  RndMeth=Nearest  InputSameDT=on
  RelationalOperator3 : RelationalOperator  Operator=>  OutDataTypeStr=boolean  ZeroCross=off  RndMeth=Nearest  InputSameDT=on
  Subtract4 : Sum  Inputs=-+  OutDataTypeStr=Inherit: Inherit via back propagation  RndMeth=Zero  InputSameDT=on
      settings: AttributesFormatString=SAT = %<SaturateOnIntegerOverflow>
  Sum : Sum  Inputs=+-  OutDataTypeStr=Inherit: Inherit via back propagation  RndMeth=Zero  InputSameDT=on
      settings: AttributesFormatString=SAT = %<SaturateOnIntegerOverflow>
  Switch{1..5} (5 alike) : Switch  Tag=GEN,Threshold;AM,Switch,2,1;CMP,Threshold;  Criteria=u2 ~= 0  RndMeth=Zero  SaturateOnIntegerOverflow=on  InputSameDT=on
      settings: AttributesFormatString=Criteria=%<Criteria>
  Timeout : Outport
      settings: AttributesFormatString=Type : %<OutDataTypeStr>\nInit :%<InitialOutput>
  Timer : Outport  Port=2
      settings: AttributesFormatString=Type : %<OutDataTypeStr>\nInit :%<InitialOutput>
  wires:
  [Timestep] -> Subtract4/2, Product3/2
  Constant6/1 -> RelationalOperator3/2
  Constant2/1 -> Product3/1
  [Timestep1] -> Sum/2, RelationalOperator2/1
  [EdgeRising] -> Switch2/2
  EdgeRising/1(Out) -> Switch1/2, Switch1/1, [EdgeRising]
  Init/1 -> EdgeRising/1(In)
  LogicalOperator/1 -> Timeout/1
  Switch1/1 -> DelayInput1/1, LogicalOperator/1
  DelayInput1/1 -> Switch1/3
  RelationalOperator1/1 -> LogicalOperator/2, Switch3/2
  Switch3/1 -> DelayInput2/1, Product2/1
  Product2/1 -> Timer/1
  Switch2/1 -> Switch3/3, RelationalOperator1/2
  Switch4/1 -> Switch2/3
  Sum/1 -> Switch4/1
  RelationalOperator2/1 -> Switch4/2
  DelayInput2/1 -> Sum/1, RelationalOperator2/2
  [Timestep1] -> RelationalOperator1/1
  Constant3/1 -> Switch3/1
  Constant1/1 -> Switch4/3
  Product3/1 -> DataTypeConversion2/1
  DataTypeConversion2/1 -> Subtract4/1
  RelationalOperator3/1 -> Switch5/2
  Subtract4/1 -> RelationalOperator3/1
  Constant5/1 -> Switch5/3
  Constant4/1 -> Switch5/1
  Switch5/1 -> [tolcons]
  [tolcons] -> Product/2
  Product/1 -> [Timestep1]
  Duration/1 -> Product1/1, RSA_DataTypeDuplicate_v01_03/2
  [tolcons] -> Product1/2
  Product1/1 -> Switch2/1
  Timestep/1 -> RSA_DataTypeDuplicate_v01_03/1, [Timestep], Product/1
  [tolcons] -> Product2/2

== system Renault_Library/Complex blocks/Timers & Counters/RSA_Timer_Conversion ==
  TIMER_TO_ADAPT : Inport
  Constant1 : Constant  Value=SAMPLE_TIME_CST  OutDataTypeStr=Inherit: Inherit via back propagation  SampleTime=-1
  Constant2 : Constant  Value=SAMPLE_TIME_CST  OutDataTypeStr=Inherit: Inherit via back propagation  SampleTime=-1
  Constant3 : Constant  Value=ZERO_CST  OutDataTypeStr=Inherit: Inherit via back propagation  SampleTime=-1
  Divide : Product  Inputs=*/  OutDataTypeStr=Inherit: Inherit via back propagation  SaturateOnIntegerOverflow=on  RndMeth=Zero
      settings: AttributesFormatString=Saturation:%<SaturateOnIntegerOverflow>
  RelationalOperator1 : RelationalOperator  Operator===  OutDataTypeStr=boolean  RndMeth=Nearest  InputSameDT=on
  Switch : Switch  Criteria=u2 ~= 0  RndMeth=Zero  InputSameDT=on
      settings: AttributesFormatString=Criteria=%<Criteria>
  TIMER_ADAPTED : Outport
  wires:
  Constant2/1 -> Divide/2
  Constant1/1 -> RelationalOperator1/1
  Constant3/1 -> RelationalOperator1/2
  RelationalOperator1/1 -> Switch/2
  Switch/1 -> TIMER_ADAPTED/1
  TIMER_TO_ADAPT/1 -> [TIMER_TO_ADAPT]
  [TIMER_TO_ADAPT] -> Switch/1
  [TIMER_TO_ADAPT] -> Divide/1
  Divide/1 -> Switch/3

== system Renault_Library/Complex blocks/Timers & Counters/RSA_Timer_Conversion1 ==
  TIMER_TO_ADAPT : Inport
  Constant1 : Constant  Value=SAMPLE_TIME_CST  OutDataTypeStr=Inherit: Inherit via back propagation  SampleTime=-1
  Constant2 : Constant  Value=SAMPLE_TIME_CST  OutDataTypeStr=Inherit: Inherit via back propagation  SampleTime=-1
  Constant3 : Constant  Value=ZERO_CST  OutDataTypeStr=uint8  SampleTime=-1
  Divide : Product  Inputs=*/  OutDataTypeStr=Inherit: Inherit via back propagation  SaturateOnIntegerOverflow=on  RndMeth=Zero
      settings: AttributesFormatString=Saturation:%<SaturateOnIntegerOverflow>
  RelationalOperator1 : RelationalOperator  Operator=>  OutDataTypeStr=boolean  RndMeth=Nearest  InputSameDT=on
  Switch : Switch  Criteria=u2 ~= 0  RndMeth=Zero  InputSameDT=on
      settings: AttributesFormatString=Criteria=%<Criteria>
  TIMER_ADAPTED : Outport
  wires:
  Divide/1 -> Switch/1
  [TIMER_TO_ADAPT] -> Divide/1
  [TIMER_TO_ADAPT] -> Switch/3
  TIMER_TO_ADAPT/1 -> [TIMER_TO_ADAPT]
  Switch/1 -> TIMER_ADAPTED/1
  RelationalOperator1/1 -> Switch/2
  Constant3/1 -> RelationalOperator1/2
  Constant1/1 -> RelationalOperator1/1
  Constant2/1 -> Divide/2

== system Renault_Library/Complex blocks/Timers & Counters/RSA_Timer_Conversion_v01_01 ==
  TIMER_TO_ADAPT : Inport
  Constant1 : Constant  Value=SAMPLE_TIME_CST  OutDataTypeStr=Inherit: Inherit via back propagation  SampleTime=-1
  Constant2 : Constant  Value=SAMPLE_TIME_CST  OutDataTypeStr=Inherit: Inherit via back propagation  SampleTime=-1
  Constant3 : Constant  Value=ZERO_CST  OutDataTypeStr=uint8  SampleTime=-1
  Divide : Product  Inputs=*/  OutDataTypeStr=Inherit: Inherit via back propagation  SaturateOnIntegerOverflow=on  RndMeth=Zero
      settings: AttributesFormatString=Saturation:%<SaturateOnIntegerOverflow>
  RelationalOperator1 : RelationalOperator  Operator===  OutDataTypeStr=boolean  RndMeth=Nearest  InputSameDT=on
  Switch : Switch  Criteria=u2 ~= 0  RndMeth=Zero  InputSameDT=on
      settings: AttributesFormatString=Criteria=%<Criteria>
  TIMER_ADAPTED : Outport
  wires:
  Constant2/1 -> Divide/2
  Constant1/1 -> RelationalOperator1/1
  Constant3/1 -> RelationalOperator1/2
  RelationalOperator1/1 -> Switch/2
  Switch/1 -> TIMER_ADAPTED/1
  TIMER_TO_ADAPT/1 -> [TIMER_TO_ADAPT]
  [TIMER_TO_ADAPT] -> Switch/1
  [TIMER_TO_ADAPT] -> Divide/1
  Divide/1 -> Switch/3

== system Renault_Library/Complex blocks/Timers & Counters/RSA_Timer_v01_04 ==
  Init : Inport
      settings: AttributesFormatString=Type : %<OutDataTypeStr>
  Duration : Inport  Port=2
      settings: AttributesFormatString=Type : %<OutDataTypeStr>
  Constant1 : Constant  Tag=AM,Constant,0,1,Value;CMP,Value;  Value=TIM1INI  OutDataTypeStr=Inherit: Inherit via back propagation  SampleTime=-1
  Constant2 : Constant  Tag=AM,Constant,0,1,Value;CMP,Value;  Value=0  OutDataTypeStr=Inherit: Inherit via back propagation  SampleTime=-1
  Constant3 : Constant  Tag=AM,Constant,0,1,Value;CMP,Value;  Value=st  OutDataTypeStr=Inherit: Inherit via back propagation  SampleTime=-1
  Constant4 : Constant  Tag=AM,Constant,0,1,Value;CMP,Value;  Value=st  OutDataTypeStr=Inherit: Inherit via back propagation  SampleTime=-1
  DataTypeConversion : DataTypeConversion  RndMeth=Zero
  DelayInput1 : UnitDelay  Description=\nStore in Global RAM  InitialCondition=timeoutinit  SampleTime=-1
      settings: AttributesFormatString=IC = %<X0>
  DelayInput2 : UnitDelay  Description=\nStore in Global RAM  InitialCondition=TIM1INI  SampleTime=-1
      settings: AttributesFormatString=IC = %<X0>
  EdgeRising : Subsystem  mask(EdgeRising){vinit=initinit}
      mask prompts: vinit "Initialisation"
      mask help: When a rising edge is used as input, the ouput is equal to one during the fall of the signal. Otherwise the output is zero.
      mask Display:
      | plot([0.2 0.4 0.4 0.6],[0.6 0.6 0.9 0.9]);
      | plot([0.2 0.4 0.4 0.4 0.6],[0.1 0.1 0.4 0.1 0.1])
  LogicalOperator : Logic  OutDataTypeStr=boolean
  RelationalOperator1 : RelationalOperator  Operator=>  OutDataTypeStr=boolean  ZeroCross=off  RndMeth=Nearest  InputSameDT=on
  RelationalOperator2 : RelationalOperator  Operator=<=  OutDataTypeStr=boolean  ZeroCross=off  RndMeth=Nearest  InputSameDT=on
  Sum : Sum  Inputs=+-  OutDataTypeStr=Inherit: Inherit via back propagation  RndMeth=Zero  SaturateOnIntegerOverflow=on  InputSameDT=on
      settings: AttributesFormatString=SAT = %<SaturateOnIntegerOverflow>
  Switch{1..4} (4 alike) : Switch  Tag=GEN,Threshold;AM,Switch,2,1;CMP,Threshold;  Criteria=u2 ~= 0  RndMeth=Zero  SaturateOnIntegerOverflow=on  InputSameDT=on
      settings: AttributesFormatString=Criteria=%<Criteria>
  Timeout : Outport
      settings: AttributesFormatString=Type : %<OutDataTypeStr>\nInit :%<InitialOutput>
  Timer : Outport  Port=2
      settings: AttributesFormatString=Type : %<OutDataTypeStr>\nInit :%<InitialOutput>
  wires:
  Constant2/1 -> Switch3/1
  Switch2/1 -> RelationalOperator1/2, Switch3/3  "X3"
  Constant1/1 -> Switch4/3
  Sum/1 -> Switch4/1
  Switch4/1 -> Switch2/3
  RelationalOperator2/1 -> Switch4/2
  Constant4/1 -> RelationalOperator1/1
  Duration/1 -> Switch2/1
  DataTypeConversion/1 -> Timer/1
  EdgeRising/1(Out) -> Switch1/2, Switch1/1, [EdgeRising]
  Init/1 -> EdgeRising/1(In)
  LogicalOperator/1 -> Timeout/1
  Switch1/1 -> DelayInput1/1, LogicalOperator/1  "Flag_of_first_initialisation"
  DelayInput1/1 -> Switch1/3
  RelationalOperator1/1 -> Switch3/2, LogicalOperator/2  "X4"
  Switch3/1 -> DelayInput2/1, DataTypeConversion/1
  Constant3/1 -> Sum/2, RelationalOperator2/1
  [EdgeRising] -> Switch2/2
  DelayInput2/1 -> Sum/1, RelationalOperator2/2  "X2"
